simulink library "inp"  (Simulink 8.2)
settings: Solver=ode45  SolverMode=SingleTasking  StartTime=0.0  StopTime=10.0  RelTol=1e-3
undefined symbols (not defined in the file or in its folder): C_TICK_TIME_S, ENUM_FAULTSTATE_FAIL, ENUM_FAULTSTATE_INDETERMINATE, ENUM_FAULTSTATE_PASS, ENUM_KEYSWITCH_ON, FALSE, KINP_ABS1CANCommuOk_flg_ovrdflg, KINP_ABS1CANCommuOk_flg_ovrdval, KINP_ABS1CANPeriodTime_s, KINP_ABS1CANRolCntOk_flg_ovrdflg, KINP_ABS1CANRolCntOk_flg_ovrdval, KINP_ABS2CANChkSumOk_flg_ovrdflg, KINP_ABS2CANChkSumOk_flg_ovrdval, KINP_ABS2CANCommuOk_flg_ovrdflg, KINP_ABS2CANCommuOk_flg_ovrdval, KINP_ABS2CANPeriodTime_s, KINP_ABS2CANRolCntOk_flg_ovrdflg, KINP_ABS2CANRolCntOk_flg_ovrdval, KINP_ABS3CANCommuOk_flg_ovrdflg, KINP_ABS3CANCommuOk_flg_ovrdval, KINP_ABS4CANCommuOk_flg_ovrdflg, KINP_ABS4CANCommuOk_flg_ovrdval, KINP_ABSActive_flg_defval, KINP_ABSActive_flg_invalid, KINP_ABSActive_flg_ovrdflg, KINP_ABSActive_flg_ovrdval, KINP_ABSActive_flg_setvalid, KINP_ABSActive_flg_srvcflg, KINP_ABSActive_flg_srvcval, KINP_ABSCANAlive_flg_ovrdflg, KINP_ABSCANAlive_flg_ovrdval, KINP_ABSCANCommuOk_flg_ovrdflg, KINP_ABSCANCommuOk_flg_ovrdval, KINP_ABSCANValidOk_flg_ovrdflg, KINP_ABSCANValidOk_flg_ovrdval, KINP_ABSFault_flg_defval, KINP_ABSFault_flg_invalid, KINP_ABSFault_flg_ovrdflg, KINP_ABSFault_flg_ovrdval, KINP_ABSFault_flg_setvalid, KINP_ABSFault_flg_srvcflg, KINP_ABSFault_flg_srvcval, KINP_AC1CANCommuOk_flg_ovrdflg, KINP_AC1CANCommuOk_flg_ovrdval, KINP_ACCANAlive_flg_ovrdflg, KINP_ACCANAlive_flg_ovrdval, KINP_ACCANCommuOk_flg_ovrdflg, KINP_ACCANCommuOk_flg_ovrdval, KINP_ACCANValidOk_flg_ovrdflg, KINP_ACCANValidOk_flg_ovrdval, KINP_ACCCancelH_flg_defval, KINP_ACCCancelH_flg_invalid, KINP_ACCCancelH_flg_ovrdflg, KINP_ACCCancelH_flg_ovrdval, KINP_ACCCancelH_flg_setvalid, KINP_ACCCancelH_flg_srvcflg, KINP_ACCCancelH_flg_srvcval, KINP_AccelPedalPosn_pct_defval, KINP_AccelPedalPosn_pct_gain, KINP_AccelPedalPosn_pct_invalid ... (+1866)
libraries: inp x192, hcu_lib x34, simulink x33
(part 1 of 21)
settings Model: Creator=WX  LastModifiedBy=13192

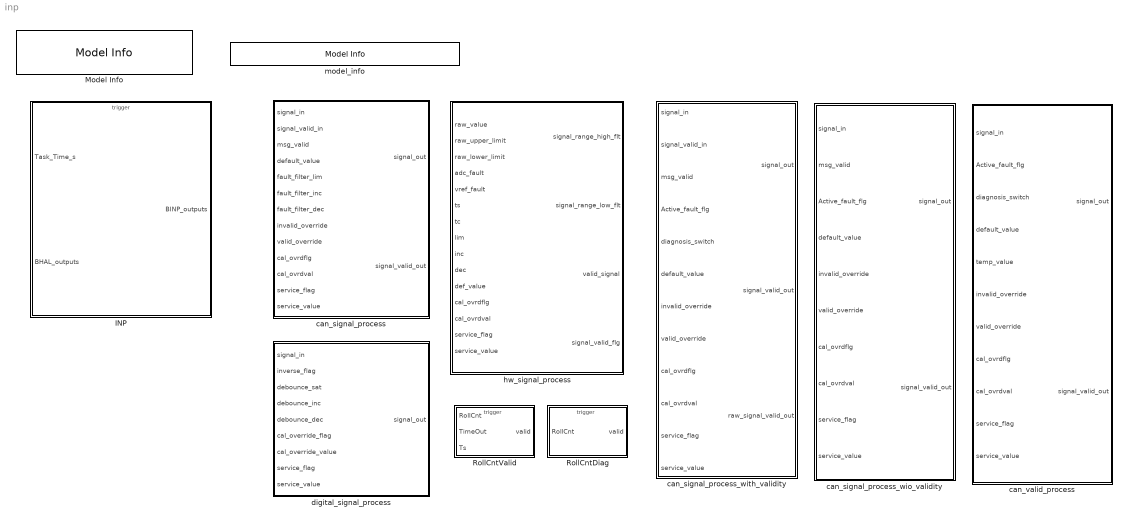
== system inp ==
  INP : Subsystem
      settings: RTWSystemCode=Nonreusable function  RTWFcnNameOpts=Use subsystem name  RTWFileNameOpts=Use subsystem name
  Model Info : simulink/Model-Wide Utilities/Model Info  InitialBlockCM=None  BlockCM=None  Frame=on  DisplayStringWithTags=|  HorizontalTextAlignment=Left  LeftAlignmentValue=0.02  SourceBlockDiagram=inp  TagMaxNumber=20
      | Model Version        : %<ModelVersion>
      | Creator                   : %<Creator>
      | Last Modified by    : %<LastModifiedBy>
  RollCntDiag : Subsystem
  RollCntValid : Subsystem
  can_signal_process : Subsystem
  can_signal_process_wio_validity : Subsystem
  can_signal_process_with_validity : Subsystem
  can_valid_process : Subsystem
  digital_signal_process : Subsystem
  hw_signal_process : Subsystem
  model_info : simulink/Model-Wide Utilities/Model Info  InitialBlockCM=None  BlockCM=None  Frame=on  DisplayStringWithTags=$Id: hcu_lib.mdl 289 2016-04-11 06:01:08Z chimotsai $  HorizontalTextAlignment=Center  LeftAlignmentValue=0.5  SourceBlockDiagram=inp  TagMaxNumber=20

== system inp/INP ==
  Task_Time_s : Inport
  BHAL_outputs : Inport  Port=2  OutDataTypeStr=Bus: BHAL_outputs
  trig_inp : TriggerPort  TriggerType=function-call
  ABS_signals : Subsystem
  AC_signals : Subsystem
  BCM_signals : Subsystem
  CAN_Message_Valid_Proc : Subsystem
  CAN_Signals_Protected : Subsystem
  CSW_signals : Subsystem
  ECM_signals : Subsystem
  EPB_signals : Subsystem
  EPM_signals : Subsystem
  EPS_signals : Subsystem
  HMI_signals : Subsystem
  HWIO_signals : Subsystem
  InputSignals : Subsystem
  LLSW_signals : Subsystem
  METER_signals : Subsystem
  TCM_signals : Subsystem
  VCU_signals : Subsystem
  BINP_outputs : Outport  OutDataTypeStr=Bus: BINP_outputs
  wires:
  [CANvalid_bus] -> ECM_signals/2(CANvalid_bus)
  CAN_Signals_Protected/1(ProtCC_bus) -> [ProtCC_bus]
  [BHAL_outputs] -> CAN_Signals_Protected/1(BHAL_outputs)
  [Task_Time_s] -> CAN_Message_Valid_Proc/2(Task_Time_s)
  CAN_Message_Valid_Proc/1(CANvalid_bus) -> [CANvalid_bus]
  [BHAL_outputs] -> LLSW_signals/1(BHAL_outputs)
  [Task_Time_s] -> InputSignals/15(Task_Time_s)
  LLSW_signals/1(LLSW_signal_bus) -> InputSignals/14(LLSW_signal_bus)
  [Task_Time_s] -> HWIO_signals/2(Task_Time_s)
  [BHAL_outputs] -> HWIO_signals/1(BHAL_outputs)
  [BHAL_outputs] -> EPM_signals/1(BHAL_outputs)
  [BHAL_outputs] -> EPS_signals/1(BHAL_outputs)
  [BHAL_outputs] -> ABS_signals/1(BHAL_outputs)
  [BHAL_outputs] -> BCM_signals/1(BHAL_outputs)
  [BHAL_outputs] -> TCM_signals/1(BHAL_outputs)
  Task_Time_s/1 -> [Task_Time_s]
  [BHAL_outputs] -> ECM_signals/1(BHAL_outputs)
  HWIO_signals/1(HWIO_signal_bus) -> InputSignals/13(HWIO_signal_bus)
  EPM_signals/1(EPM_signal_bus) -> InputSignals/12(EPM_signal_bus)
  EPS_signals/1(EPS_signal_bus) -> InputSignals/5(EPS_signal_bus)
  ABS_signals/1(ABS_signal_bus) -> InputSignals/4(ABS_signal_bus)
  BCM_signals/1(BCM_signal_bus) -> InputSignals/3(BCM_signal_bus)
  TCM_signals/1(TCM_signal_bus) -> InputSignals/2(TCM_signal_bus)
  ECM_signals/1(ECM_signal_bus) -> InputSignals/1(ECM_signal_bus)
  InputSignals/1(BINP_outputs) -> BINP_outputs/1
  BHAL_outputs/1 -> [BHAL_outputs], CAN_Message_Valid_Proc/1(BHAL_outputs)
  [CANvalid_bus] -> TCM_signals/2(CANvalid_bus)
  [CANvalid_bus] -> BCM_signals/2(CANvalid_bus)
  [CANvalid_bus] -> ABS_signals/2(CANvalid_bus)
  [CANvalid_bus] -> CAN_Signals_Protected/2(CANvalid_bus)
  [CANvalid_bus] -> EPS_signals/2(CANvalid_bus)
  [CANvalid_bus] -> EPM_signals/2(CANvalid_bus)
  [CANvalid_bus] -> InputSignals/17(CANvalid_bus)
  [ProtCC_bus] -> InputSignals/16(ProtCC_bus)
  [BHAL_outputs] -> METER_signals/1(BHAL_outputs)
  [CANvalid_bus] -> METER_signals/2(CANvalid_bus)
  [BHAL_outputs] -> AC_signals/1(BHAL_outputs)
  [CANvalid_bus] -> AC_signals/2(CANvalid_bus)
  [BHAL_outputs] -> EPB_signals/1(BHAL_outputs)
  [CANvalid_bus] -> EPB_signals/2(CANvalid_bus)
  METER_signals/1(METER_signal_bus) -> InputSignals/6(METER_signal_bus)
  AC_signals/1(AC_signal_bus) -> InputSignals/7(AC_signal_bus)
  EPB_signals/1(EPB_signal_bus) -> InputSignals/8(EPB_signal_bus)
  [BHAL_outputs] -> VCU_signals/1(BHAL_outputs)
  [CANvalid_bus] -> VCU_signals/2(CANvalid_bus)
  VCU_signals/1(VCU_signal_bus) -> InputSignals/10(VCU_signal_bus)
  [BHAL_outputs] -> HMI_signals/1(BHAL_outputs)
  [CANvalid_bus] -> HMI_signals/2(CANvalid_bus)
  HMI_signals/1(HMI_signal_bus) -> InputSignals/11(HMI_signal_bus)
  [BHAL_outputs] -> CSW_signals/1(BHAL_outputs)
  [CANvalid_bus] -> CSW_signals/2(CANvalid_bus)
  CSW_signals/1(CSW_signal_bus) -> InputSignals/9(CSW_signal_bus)

== system inp/RollCntDiag ==
  RollCnt : Inport
  Sampler : TriggerPort  TriggerType=function-call
  Constant : Constant  Value=FALSE
  Constant1 : Constant  Value=TRUE
  Logical Operator : Logic  Operator=NOT  AllPortsSameDT=off  OutDataTypeStr=boolean
  Relational Operator1 : RelationalOperator  Operator===  OutDataTypeStr=boolean
  Switch : Switch  Criteria=u2 ~= 0
  Unit Delay1 : UnitDelay  SampleTime=-1
  Unit Delay2 : UnitDelay  InitialCondition=TRUE  SampleTime=-1
  valid : Outport
  wires:
  Relational Operator1/1 -> Logical Operator/1
  Unit Delay1/1 -> Relational Operator1/1
  RollCnt/1 -> Relational Operator1/2, Unit Delay1/1
  Logical Operator/1 -> Switch/3
  Switch/1 -> valid/1
  Constant/1 -> Unit Delay2/1
  Unit Delay2/1 -> Switch/2
  Constant1/1 -> Switch/1

== system inp/RollCntValid ==
  RollCnt : Inport
  TimeOut : Inport  Port=2
  Ts : Inport  Port=3
  Sampler : TriggerPort  TriggerType=function-call
  Add : Sum
  RESET : Constant  Value=0  OutDataTypeStr=single
  Relational Operator1 : RelationalOperator  Operator===  OutDataTypeStr=boolean
  Relational Operator2 : RelationalOperator  Operator=<  OutDataTypeStr=boolean
  Switch : Switch  Criteria=u2 ~= 0
  Unit Delay : UnitDelay  SampleTime=-1
  Unit Delay1 : UnitDelay  SampleTime=-1
  valid : Outport
  wires:
  Ts/1 -> Add/2
  Add/1 -> Switch/1
  Unit Delay/1 -> Add/1
  RollCnt/1 -> Unit Delay1/1, Relational Operator1/2
  Unit Delay1/1 -> Relational Operator1/1
  Switch/1 -> Unit Delay/1, Relational Operator2/1
  Relational Operator1/1 -> Switch/2
  RESET/1 -> Switch/3
  TimeOut/1 -> Relational Operator2/2
  Relational Operator2/1 -> valid/1

== system inp/can_signal_process ==
  signal_in : Inport
  signal_valid_in : Inport  Port=2
  msg_valid : Inport  Port=3
  default_value : Inport  Port=4
  fault_filter_lim : Inport  Port=5
  fault_filter_inc : Inport  Port=6
  fault_filter_dec : Inport  Port=7
  invalid_override : Inport  Port=8
  valid_override : Inport  Port=9
  cal_ovrdflg : Inport  Port=10
  cal_ovrdval : Inport  Port=11
  service_flag : Inport  Port=12
  service_value : Inport  Port=13
  Logical Operator1 : Logic  Operator=NOT  AllPortsSameDT=off  OutDataTypeStr=boolean
  Logical Operator2 : Logic  AllPortsSameDT=off  OutDataTypeStr=boolean
  SRS_HCU_INP_002 : simulink/Model-Wide Utilities/DocBlock  DocumentType=Text
  override signal : hcu_lib/override signal
  signal validity checking : hcu_lib/sig_valid_check
  signal_out : Outport
  signal_valid_out : Outport  Port=2
  wires:
  override signal/2 -> signal_valid_out/1
  override signal/1 -> signal_out/1
  msg_valid/1 -> Logical Operator2/2
  signal_in/1 -> signal validity checking/1
  signal_valid_in/1 -> Logical Operator2/1
  Logical Operator2/1 -> signal validity checking/2
  invalid_override/1 -> signal validity checking/3
  Logical Operator1/1 -> override signal/1  "signal_valid_flg_raw"
  service_flag/1 -> override signal/3
  service_value/1 -> override signal/4
  cal_ovrdflg/1 -> override signal/5
  cal_ovrdval/1 -> override signal/6
  valid_override/1 -> signal validity checking/4
  default_value/1 -> signal validity checking/5
  fault_filter_lim/1 -> signal validity checking/6
  fault_filter_inc/1 -> signal validity checking/7
  fault_filter_dec/1 -> signal validity checking/8
  signal validity checking/1 -> Logical Operator1/1  "fault_flg"
  signal validity checking/2 -> override signal/2  "valid_flg"

== system inp/can_signal_process_wio_validity ==
  signal_in : Inport
  msg_valid : Inport  Port=2
  Active_fault_flg : Inport  Port=3
  default_value : Inport  Port=4
  invalid_override : Inport  Port=5
  valid_override : Inport  Port=6
  cal_ovrdflg : Inport  Port=7
  cal_ovrdval : Inport  Port=8
  service_flag : Inport  Port=9
  service_value : Inport  Port=10
  CAN signal validity checking no diag : hcu_lib/CAN signal validity checking no diag
  Logical Operator1 : Logic  Operator=NOT  AllPortsSameDT=off  OutDataTypeStr=boolean
  override signal : hcu_lib/override signal
  signal_out : Outport
  signal_valid_out : Outport  Port=2
  wires:
  override signal/2 -> signal_valid_out/1
  override signal/1 -> signal_out/1
  msg_valid/1 -> CAN signal validity checking no diag/2
  signal_in/1 -> CAN signal validity checking no diag/1
  invalid_override/1 -> CAN signal validity checking no diag/3
  Logical Operator1/1 -> override signal/1  "signal_valid_flg_raw"
  service_flag/1 -> override signal/3
  service_value/1 -> override signal/4
  cal_ovrdflg/1 -> override signal/5
  cal_ovrdval/1 -> override signal/6
  valid_override/1 -> CAN signal validity checking no diag/4
  default_value/1 -> CAN signal validity checking no diag/5
  Active_fault_flg/1 -> CAN signal validity checking no diag/6
  CAN signal validity checking no diag/2 -> override signal/2  "signal_proc"
  CAN signal validity checking no diag/1 -> Logical Operator1/1  "fault_flag"

== system inp/can_signal_process_with_validity ==
  signal_in : Inport
  signal_valid_in : Inport  Port=2
  msg_valid : Inport  Port=3
  Active_fault_flg : Inport  Port=4
  diagnosis_switch : Inport  Port=5
  default_value : Inport  Port=6
  invalid_override : Inport  Port=7
  valid_override : Inport  Port=8
  cal_ovrdflg : Inport  Port=9
  cal_ovrdval : Inport  Port=10
  service_flag : Inport  Port=11
  service_value : Inport  Port=12
  CAN signal validity checking : hcu_lib/CAN signal validity checking
  Logical Operator1 : Logic  Operator=NOT  AllPortsSameDT=off  OutDataTypeStr=boolean
  Logical Operator2 : Logic  Operator=NOT  AllPortsSameDT=off  OutDataTypeStr=boolean
  override signal : hcu_lib/override signal
  signal_out : Outport
  signal_valid_out : Outport  Port=2
  raw_signal_valid_out : Outport  Port=3
  wires:
  CAN signal validity checking/1 -> Logical Operator2/1
  CAN signal validity checking/2 -> Logical Operator1/1  "fault_flag"
  CAN signal validity checking/3 -> override signal/2  "signal_proc"
  Active_fault_flg/1 -> CAN signal validity checking/7
  default_value/1 -> CAN signal validity checking/6
  valid_override/1 -> CAN signal validity checking/5
  cal_ovrdval/1 -> override signal/6
  cal_ovrdflg/1 -> override signal/5
  service_value/1 -> override signal/4
  service_flag/1 -> override signal/3
  Logical Operator1/1 -> override signal/1  "signal_valid_flg_raw"
  invalid_override/1 -> CAN signal validity checking/4
  signal_valid_in/1 -> CAN signal validity checking/2
  signal_in/1 -> CAN signal validity checking/1
  msg_valid/1 -> CAN signal validity checking/3
  override signal/1 -> signal_out/1
  override signal/2 -> signal_valid_out/1
  diagnosis_switch/1 -> CAN signal validity checking/8
  Logical Operator2/1 -> raw_signal_valid_out/1

== system inp/can_valid_process ==
  signal_in : Inport
  Active_fault_flg : Inport  Port=2
  diagnosis_switch : Inport  Port=3
  default_value : Inport  Port=4
  temp_value : Inport  Port=5
  invalid_override : Inport  Port=6
  valid_override : Inport  Port=7
  cal_ovrdflg : Inport  Port=8
  cal_ovrdval : Inport  Port=9
  service_flag : Inport  Port=10
  service_value : Inport  Port=11
  Logical Operator1 : Logic  Operator=NOT  AllPortsSameDT=off  OutDataTypeStr=boolean
  override signal : hcu_lib/override signal
  signal validity checking with temp value : hcu_lib/signal validity checking with temp value
  signal_out : Outport
  signal_valid_out : Outport  Port=2
  wires:
  signal validity checking with temp value/2 -> override signal/2  "signal_proc"
  signal validity checking with temp value/1 -> Logical Operator1/1  "fault_flg"
  Active_fault_flg/1 -> signal validity checking with temp value/7
  default_value/1 -> signal validity checking with temp value/6
  valid_override/1 -> signal validity checking with temp value/4
  cal_ovrdval/1 -> override signal/6
  cal_ovrdflg/1 -> override signal/5
  service_value/1 -> override signal/4
  service_flag/1 -> override signal/3
  Logical Operator1/1 -> override signal/1  "signal_valid_flg_raw"
  invalid_override/1 -> signal validity checking with temp value/3
  signal_in/1 -> signal validity checking with temp value/2, signal validity checking with temp value/1
  override signal/1 -> signal_out/1
  override signal/2 -> signal_valid_out/1
  temp_value/1 -> signal validity checking with temp value/5
  diagnosis_switch/1 -> signal validity checking with temp value/8

== system inp/digital_signal_process ==
  signal_in : Inport
  inverse_flag : Inport  Port=2
  debounce_sat : Inport  Port=3
  debounce_inc : Inport  Port=4
  debounce_dec : Inport  Port=5
  cal_override_flag : Inport  Port=6
  cal_override_value : Inport  Port=7
  service_flag : Inport  Port=8
  service_value : Inport  Port=9
  Ground : Ground
  Logical Operator : Logic  Operator=NOT  Inputs=1  AllPortsSameDT=off  OutDataTypeStr=boolean
  Switch1 : Switch  Criteria=u2 ~= 0  Threshold=0.5
  Terminator : Terminator
  Terminator1 : Terminator
  override_signal : hcu_lib/override signal
  resetting fault filter : hcu_lib/fault_filter_reset
  signal_out : Outport  OutDataTypeStr=boolean
  wires:
  debounce_dec/1 -> resetting fault filter/4
  debounce_inc/1 -> resetting fault filter/3
  debounce_sat/1 -> resetting fault filter/2
  override_signal/1 -> signal_out/1
  resetting fault filter/2 -> Terminator/1
  override_signal/2 -> Terminator1/1
  resetting fault filter/1 -> override_signal/2
  Ground/1 -> override_signal/1
  service_flag/1 -> override_signal/3
  service_value/1 -> override_signal/4
  cal_override_flag/1 -> override_signal/5
  cal_override_value/1 -> override_signal/6
  inverse_flag/1 -> Switch1/2
  signal_in/1 -> Switch1/3, Logical Operator/1
  Switch1/1 -> resetting fault filter/1
  Logical Operator/1 -> Switch1/1

== system inp/hw_signal_process ==
  raw_value : Inport
  raw_upper_limit : Inport  Port=2
  raw_lower_limit : Inport  Port=3
  adc_fault : Inport  Port=4
  vref_fault : Inport  Port=5
  ts : Inport  Port=6
  tc : Inport  Port=7
  lim : Inport  Port=8
  inc : Inport  Port=9
  dec : Inport  Port=10
  def_value : Inport  Port=11
  cal_ovrdflg : Inport  Port=12
  cal_ovrdval : Inport  Port=13
  service_flag : Inport  Port=14
  service_value : Inport  Port=15
  Logical Operator2 : Logic  Operator=NOT  AllPortsSameDT=off  OutDataTypeStr=boolean
  Relational Operator : RelationalOperator  Operator=<  OutDataTypeStr=boolean
  Relational Operator1 : RelationalOperator  Operator=<  OutDataTypeStr=boolean
  SRS_HCU_INP_004 : simulink/Model-Wide Utilities/DocBlock  DocumentType=Text
  override signal : hcu_lib/override signal
  sig_valid_check : Subsystem  RTWFcnName=sig_valid_check
      settings: RTWFcnNameOpts=User specified
  signal_filter : Subsystem
  signal_range_high_flt : Outport
  signal_range_low_flt : Outport  Port=2
  valid_signal : Outport  Port=3
  signal_valid_flg : Outport  Port=4
  wires:
  Logical Operator2/1 -> override signal/1
  ts/1 -> signal_filter/3(Ts)
  tc/1 -> signal_filter/2(Tc)
  raw_value/1 -> [raw_value], signal_filter/1(raw_input)
  Relational Operator/1 -> sig_valid_check/4(range_high_ifault_flg)
  [raw_value] -> Relational Operator1/1, Relational Operator/2
  def_value/1 -> sig_valid_check/9(def_value)
  dec/1 -> sig_valid_check/8(dec)
  inc/1 -> sig_valid_check/7(inc)
  lim/1 -> sig_valid_check/6(lim)
  raw_lower_limit/1 -> Relational Operator1/2
  raw_upper_limit/1 -> Relational Operator/1
  sig_valid_check/3(fault_flg) -> Logical Operator2/1
  signal_filter/1(raw_input_filt) -> sig_valid_check/1(input_signal_filt)
  Relational Operator1/1 -> sig_valid_check/5(range_low_ifault_flg)
  sig_valid_check/1(range_high_flt) -> signal_range_high_flt/1
  sig_valid_check/2(range_low_flt) -> signal_range_low_flt/1
  service_flag/1 -> override signal/3
  service_value/1 -> override signal/4
  cal_ovrdflg/1 -> override signal/5
  cal_ovrdval/1 -> override signal/6
  sig_valid_check/4(valid_signal) -> override signal/2
  override signal/1 -> valid_signal/1
  override signal/2 -> signal_valid_flg/1
  adc_fault/1 -> sig_valid_check/2(adc_fault)
  vref_fault/1 -> sig_valid_check/3(vref_fault)

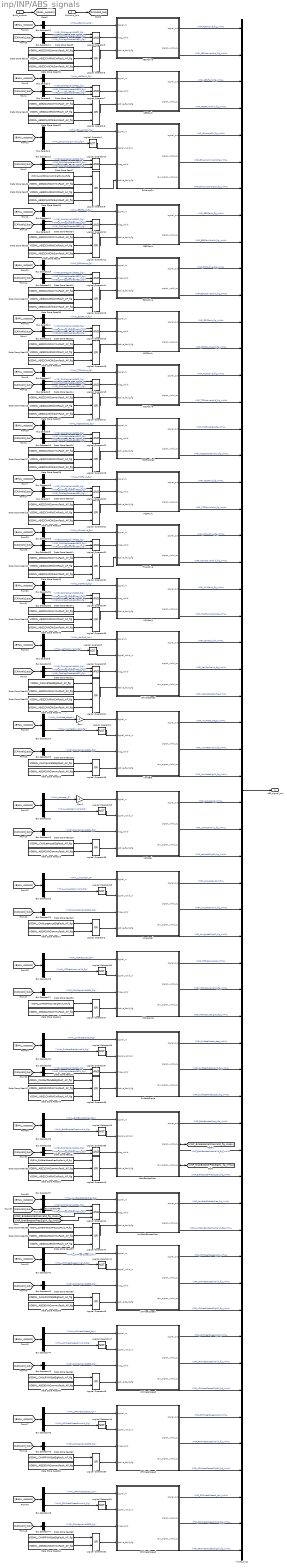
== system inp/INP/ABS_signals ==
  BHAL_outputs : Inport  OutDataTypeStr=Bus: BHAL_outputs
  CANvalid_bus : Inport  Port=2
  ABSActive : Subsystem
      settings: RTWSystemCode=Inline
  ABSFault : Subsystem
      settings: RTWSystemCode=Inline
  BrakBoosterPres : Subsystem
      settings: RTWSystemCode=Inline
  BrkLampOn : Subsystem
      settings: RTWSystemCode=Inline
  Bus Selector1 : BusSelector  OutputSignals=VHAL_ABSFault_flg
  Bus Selector10 : BusSelector  OutputSignals=VHAL_ABSActive_flg
  Bus Selector{3,5,7,9,11..12,16,18,20..21,30,32} (12 alike) : BusSelector  OutputSignals=VINP_CANMsgValidABS2_flg,VINP_CANMsgUpdatedABS2_flg,VINP_CANMsgCheckedABS2_flg
  Bus Selector{13,23,25,27} (4 alike) : BusSelector  OutputSignals=VINP_CANMsgValidABS4_flg
  Bus Selector14 : BusSelector  OutputSignals=VHAL_EBDFault_flg
  Bus Selector15 : BusSelector  OutputSignals=VHAL_ESCActive_flg
  Bus Selector17 : BusSelector  OutputSignals=VHAL_TCSActive_flg
  Bus Selector19 : BusSelector  OutputSignals=VHAL_TCSFault_flg
  Bus Selector2 : BusSelector  OutputSignals=VHAL_BrkLampOn_flg,VHAL_BrkLampSigInvalid_flg
  Bus Selector22 : BusSelector  OutputSignals=VHAL_LatAccel_G,VHAL_LatAccelInvalid_flg
  Bus Selector24 : BusSelector  OutputSignals=VHAL_YawRate_degps,VHAL_YawRateInvalid_flg
  Bus Selector26 : BusSelector  OutputSignals=VHAL_LongAccel_G,VHAL_LongAccelInvalid_flg
  Bus Selector28 : BusSelector  OutputSignals=VHAL_MCPressure_bar,VHAL_MCPressureInvalid_flg
  Bus Selector29 : BusSelector  OutputSignals=VHAL_HSAActive_flg
  Bus Selector31 : BusSelector  OutputSignals=VHAL_HSAFault_flg
  Bus Selector33 : BusSelector  OutputSignals=VINP_CANMsgValidABS1_flg,VINP_CANMsgUpdatedABS1_flg
  Bus Selector34 : BusSelector  OutputSignals=VHAL_SurfaceGrade_deg,VHAL_SurfaceGradeInvalid_flg
  Bus Selector35 : BusSelector  OutputSignals=VINP_CANMsgValidABS1_flg,VINP_CANMsgUpdatedABS1_flg
  Bus Selector36 : BusSelector  OutputSignals=VHAL_BrakBoosterPres_kPa,VHAL_BrakBoosterPresInvalid_flg
  Bus Selector37 : BusSelector  OutputSignals=VHAL_LowBrakBoosterPres_flg
  Bus Selector38 : BusSelector  OutputSignals=VINP_CANMsgValidABS1_flg,VINP_CANMsgUpdatedABS1_flg
  Bus Selector4 : BusSelector  OutputSignals=VHAL_ESCFault_flg
  Bus Selector{40,43,45,47} (4 alike) : BusSelector  OutputSignals=VINP_CANMsgValidABS3_flg
  Bus Selector42 : BusSelector  OutputSignals=VHAL_LFWheelSpeed_kph,VHAL_LFWheelSpeedInvalid_flg
  Bus Selector44 : BusSelector  OutputSignals=VHAL_LRWheelSpeed_kph,VHAL_LRWheelSpeedInvalid_flg
  Bus Selector46 : BusSelector  OutputSignals=VHAL_RFWheelSpeed_kph,VHAL_RFWheelSpeedInvalid_flg
  Bus Selector48 : BusSelector  OutputSignals=VHAL_RRWheelSpeed_kph,VHAL_RRWheelSpeedInvalid_flg
  Bus Selector6 : BusSelector  OutputSignals=VHAL_TCSEnabled_flg
  Bus Selector8 : BusSelector  OutputSignals=VHAL_VehSpd_kph,VHAL_VehSpdInvalid_flg
  Data Store Read{1..2,5,8,11,13,15,17,19..20,42,45} (12 alike) : DataStoreRead  DataStoreName=VDDML_ABS2CANRolCntFault_AF_flg
  Data Store Read{3..4,6,10,12,14,16,18,21,34,41,44} (12 alike) : DataStoreRead  DataStoreName=VDDML_ABS2CANCommuFault_AF_flg
  Data Store Read22 : DataStoreRead  DataStoreName=VDDML_CANVehSpdSigFault_AF_flg
  Data Store Read{23..25,27} (4 alike) : DataStoreRead  DataStoreName=VDDML_ABS4CANCommuFault_AF_flg
  Data Store Read26 : DataStoreRead  DataStoreName=VDDML_CANYawRateSigFault_AF_flg
  Data Store Read28 : DataStoreRead  DataStoreName=VDDML_CANLatAccelSigFault_AF_flg
  Data Store Read29 : DataStoreRead  DataStoreName=VDDML_CANLongAccelSigFault_AF_flg
  Data Store Read30 : DataStoreRead  DataStoreName=VDDML_CANMCPressureSigFault_AF_flg
  Data Store Read{9,31..33,35..40,43,46} (12 alike) : DataStoreRead  DataStoreName=VDDML_ABS2CANChkSumFault_AF_flg
  Data Store Read47 : DataStoreRead  DataStoreName=VDDML_ABS1CANCommuFault_AF_flg
  Data Store Read48 : DataStoreRead  DataStoreName=VDDML_CANSurfGradeSigFault_AF_flg
  Data Store Read49 : DataStoreRead  DataStoreName=VDDML_ABS1CANRolCntFault_AF_flg
  Data Store Read50 : DataStoreRead  DataStoreName=VDDML_ABS1CANCommuFault_AF_flg
  Data Store Read51 : DataStoreRead  DataStoreName=VDDML_CANBrakBoostPresSigFault_AF_flg
  Data Store Read52 : DataStoreRead  DataStoreName=VDDML_ABS1CANRolCntFault_AF_flg
  Data Store Read53 : DataStoreRead  DataStoreName=VDDML_CANBrakBoostPresSigFault_AF_flg
  Data Store Read54 : DataStoreRead  DataStoreName=VDDML_ABS1CANCommuFault_AF_flg
  Data Store Read55 : DataStoreRead  DataStoreName=VDDML_ABS1CANRolCntFault_AF_flg
  Data Store Read{58,60,62,64} (4 alike) : DataStoreRead  DataStoreName=VDDML_ABS3CANCommuFault_AF_flg
  Data Store Read59 : DataStoreRead  DataStoreName=VDDML_CANLFWhlSpdSigFault_AF_flg
  Data Store Read61 : DataStoreRead  DataStoreName=VDDML_CANLRWhlSpdSigFault_AF_flg
  Data Store Read63 : DataStoreRead  DataStoreName=VDDML_CANRFWhlSpdSigFault_AF_flg
  Data Store Read65 : DataStoreRead  DataStoreName=VDDML_CANRRWhlSpdSigFault_AF_flg
  Data Store Read7 : DataStoreRead  DataStoreName=VDDML_CANBrkLampOnSigFault_AF_flg
  EBDFault : Subsystem
      settings: RTWSystemCode=Inline
  ESCActive : Subsystem
      settings: RTWSystemCode=Inline
  ESCFault : Subsystem
      settings: RTWSystemCode=Inline
  Gain : Gain  Gain=-1
  Gain1 : Gain  Gain=-1
  HSAActive : Subsystem
      settings: RTWSystemCode=Inline
  HSAFault : Subsystem
      settings: RTWSystemCode=Inline
  LFWheelSpeed : Subsystem
      settings: RTWSystemCode=Inline
  LRWheelSpeed : Subsystem
      settings: RTWSystemCode=Inline
  LatAccel : Subsystem
      settings: RTWSystemCode=Inline
  Logical Operator{1,3..4,25,28..30,36,44,47,49,51} (12 alike) : Logic  Tag=struct('inputs','1','comment',struct('text','','placement',1),'logdata',struct('maxnumvals',inf,'plotchannels',[],'stepsize',0,'downsampling',1,'loggingmode',3,'channels',1),'operator','NOT','output',struct('class','default','type','Bool8','description','','address','','unit','','name','$S_$B'),'date',731430.46749816)  Operator=NOT  Inputs=1
  Logical Operator{7,9..10,12,14,16,18,20,22..23,32,34} (12 alike) : Logic  Tag=struct('inputs','1','comment',struct('text','','placement',1),'logdata',struct('maxnumvals',inf,'plotchannels',[],'stepsize',0,'downsampling',1,'loggingmode',3,'channels',1),'operator','NOT','output',struct('class','default','type','Bool8','description','','address','','unit','','name','$S_$B'),'date',731430.46749816)  Inputs=3
  Logical Operator{5..6,11,13,15,17,19,21,31,33,38,40..41} (13 alike) : Logic  Tag=struct('inputs','1','comment',struct('text','','placement',1),'logdata',struct('maxnumvals',inf,'plotchannels',[],'stepsize',0,'downsampling',1,'loggingmode',3,'channels',1),'operator','NOT','output',struct('class','default','type','Bool8','description','','address','','unit','','name','$S_$B'),'date',731430.46749816)  Operator=OR  Inputs=3
  Logical Operator{2,26..27,35,46,48,50,52} (8 alike) : Logic  Tag=struct('inputs','1','comment',struct('text','','placement',1),'logdata',struct('maxnumvals',inf,'plotchannels',[],'stepsize',0,'downsampling',1,'loggingmode',3,'channels',1),'operator','NOT','output',struct('class','default','type','Bool8','description','','address','','unit','','name','$S_$B'),'date',731430.46749816)  Operator=OR
  Logical Operator24 : Logic  Tag=struct('inputs','1','comment',struct('text','','placement',1),'logdata',struct('maxnumvals',inf,'plotchannels',[],'stepsize',0,'downsampling',1,'loggingmode',3,'channels',1),'operator','NOT','output',struct('class','default','type','Bool8','description','','address','','unit','','name','$S_$B'),'date',731430.46749816)  Operator=OR  Inputs=4
  Logical Operator37 : Logic  Tag=struct('inputs','1','comment',struct('text','','placement',1),'logdata',struct('maxnumvals',inf,'plotchannels',[],'stepsize',0,'downsampling',1,'loggingmode',3,'channels',1),'operator','NOT','output',struct('class','default','type','Bool8','description','','address','','unit','','name','$S_$B'),'date',731430.46749816)
  Logical Operator39 : Logic  Tag=struct('inputs','1','comment',struct('text','','placement',1),'logdata',struct('maxnumvals',inf,'plotchannels',[],'stepsize',0,'downsampling',1,'loggingmode',3,'channels',1),'operator','NOT','output',struct('class','default','type','Bool8','description','','address','','unit','','name','$S_$B'),'date',731430.46749816)
  Logical Operator42 : Logic  Tag=struct('inputs','1','comment',struct('text','','placement',1),'logdata',struct('maxnumvals',inf,'plotchannels',[],'stepsize',0,'downsampling',1,'loggingmode',3,'channels',1),'operator','NOT','output',struct('class','default','type','Bool8','description','','address','','unit','','name','$S_$B'),'date',731430.46749816)  Inputs=4
  Logical Operator8 : Logic  Tag=struct('inputs','1','comment',struct('text','','placement',1),'logdata',struct('maxnumvals',inf,'plotchannels',[],'stepsize',0,'downsampling',1,'loggingmode',3,'channels',1),'operator','NOT','output',struct('class','default','type','Bool8','description','','address','','unit','','name','$S_$B'),'date',731430.46749816)  Operator=OR  Inputs=4
  LongAccel : Subsystem
      settings: RTWSystemCode=Inline
  LowBrakBoosterPres : Subsystem
      settings: RTWSystemCode=Inline
  MCPressure : Subsystem
      settings: RTWSystemCode=Inline
  RFWheelSpeed : Subsystem
      settings: RTWSystemCode=Inline
  RRWheelSpeed : Subsystem
      settings: RTWSystemCode=Inline
  SurfaceGrade : Subsystem
      settings: RTWSystemCode=Inline
  TCSActive : Subsystem
      settings: RTWSystemCode=Inline
  TCSEnabled : Subsystem
      settings: RTWSystemCode=Inline
  TCSFault : Subsystem
      settings: RTWSystemCode=Inline
  VehicleSpeed : Subsystem
      settings: RTWSystemCode=Inline
  YawRate : Subsystem
      settings: RTWSystemCode=Inline
  inputs_bus2 : BusCreator  Inputs=58
  ABS_signal_bus : Outport
  wires:
  inputs_bus2/1 -> ABS_signal_bus/1
  BHAL_outputs/1 -> [BHAL_outputs]
  Bus Selector11/1 -> Logical Operator9/1  "<VINP_CANMsgValidABS2_flg>"
  [CANvalid_bus] -> Bus Selector11/1
  Bus Selector10/1 -> ABSActive/1(signal_in)  "<VHAL_ABSActive_flg>"
  [BHAL_outputs] -> Bus Selector10/1
  Logical Operator5/1 -> ABSActive/3(Active_fault_flg)
  ABSActive/1(signal_out) -> inputs_bus2/1  "VINP_ABSActive_flg_InHAL"
  ABSActive/2(signal_valid_out) -> inputs_bus2/2  "VINP_ABSActiveValid_flg_InHAL"
  Bus Selector1/1 -> ABSFault/1(signal_in)  "<VHAL_ABSFault_flg>"
  [BHAL_outputs] -> Bus Selector1/1
  ABSFault/1(signal_out) -> inputs_bus2/3  "VINP_ABSFault_flg_InHAL"
  ABSFault/2(signal_valid_out) -> inputs_bus2/4  "VINP_ABSFaultValid_flg_InHAL"
  Bus Selector2/1 -> BrkLampOn/1(signal_in)  "<VHAL_BrkLampOn_flg>"
  [BHAL_outputs] -> Bus Selector2/1
  Logical Operator1/1 -> BrkLampOn/2(signal_valid_in)
  BrkLampOn/1(signal_out) -> inputs_bus2/5  "VINP_BrkLampOn_flg_InHAL"
  BrkLampOn/2(signal_valid_out) -> inputs_bus2/6  "VINP_BrkLampOnValid_flg_InHAL"
  Bus Selector2/2 -> Logical Operator1/1  "<VHAL_BrkLampSigInvalid_flg>"
  Bus Selector14/1 -> EBDFault/1(signal_in)  "<VHAL_EBDFault_flg>"
  [BHAL_outputs] -> Bus Selector14/1
  EBDFault/1(signal_out) -> inputs_bus2/8  "VINP_EBDFault_flg_InHAL"
  EBDFault/2(signal_valid_out) -> inputs_bus2/9  "VINP_EBDFaultValid_flg_InHAL"
  Bus Selector15/1 -> ESCActive/1(signal_in)  "<VHAL_ESCActive_flg>"
  [BHAL_outputs] -> Bus Selector15/1
  ESCActive/1(signal_out) -> inputs_bus2/10  "VINP_ESCActive_flg_InHAL"
  ESCActive/2(signal_valid_out) -> inputs_bus2/11  "VINP_ESCActiveValid_flg_InHAL"
  Bus Selector4/1 -> ESCFault/1(signal_in)  "<VHAL_ESCFault_flg>"
  [BHAL_outputs] -> Bus Selector4/1
  ESCFault/1(signal_out) -> inputs_bus2/12  "VINP_ESCFault_flg_InHAL"
  ESCFault/2(signal_valid_out) -> inputs_bus2/13  "VINP_ESCFaultValid_flg_InHAL"
  Bus Selector17/1 -> TCSActive/1(signal_in)  "<VHAL_TCSActive_flg>"
  [BHAL_outputs] -> Bus Selector17/1
  TCSActive/1(signal_out) -> inputs_bus2/14  "VINP_TCSActive_flg_InHAL"
  TCSActive/2(signal_valid_out) -> inputs_bus2/15  "VINP_TCSActiveValid_flg_InHAL"
  Bus Selector6/1 -> TCSEnabled/1(signal_in)  "<VHAL_TCSEnabled_flg>"
  [BHAL_outputs] -> Bus Selector6/1
  TCSEnabled/1(signal_out) -> inputs_bus2/16  "VINP_TCSEnabled_flg_InHAL"
  TCSEnabled/2(signal_valid_out) -> inputs_bus2/17  "VINP_TCSEnabledValid_flg_InHAL"
  Bus Selector19/1 -> TCSFault/1(signal_in)  "<VHAL_TCSFault_flg>"
  [BHAL_outputs] -> Bus Selector19/1
  TCSFault/1(signal_out) -> inputs_bus2/18  "VINP_TCSFault_flg_InHAL"
  TCSFault/2(signal_valid_out) -> inputs_bus2/19  "VINP_TCSFaultValid_flg_InHAL"
  VehicleSpeed/1(signal_out) -> inputs_bus2/24  "VINP_VehSpd_kph_InHAL"
  VehicleSpeed/2(signal_valid_out) -> inputs_bus2/25  "VINP_VehSpdValid_flg_InHAL"
  Bus Selector8/2 -> Logical Operator3/1  "<VHAL_VehSpdInvalid_flg>"
  [BHAL_outputs] -> Bus Selector8/1
  Bus Selector8/1 -> VehicleSpeed/1(signal_in)  "<VHAL_VehSpd_kph>"
  Logical Operator3/1 -> VehicleSpeed/2(signal_valid_in)
  Bus Selector23/1 -> YawRate/3(msg_valid)  "<VINP_CANMsgValidABS4_flg>"
  [CANvalid_bus] -> Bus Selector23/1
  YawRate/1(signal_out) -> inputs_bus2/27  "VINP_YawRate_degps_InHAL"
  YawRate/2(signal_valid_out) -> inputs_bus2/28  "VINP_YawRateValid_flg_InHAL"
  Bus Selector24/2 -> Logical Operator4/1  "<VHAL_YawRateInvalid_flg>"
  [BHAL_outputs] -> Bus Selector24/1
  Logical Operator4/1 -> YawRate/2(signal_valid_in)
  Bus Selector24/1 -> Gain/1  "<VHAL_YawRate_degps>"
  CANvalid_bus/1 -> [CANvalid_bus]
  Logical Operator9/1 -> ABSActive/2(msg_valid)
  Bus Selector11/2 -> Logical Operator9/2  "<VINP_CANMsgUpdatedABS2_flg>"
  Data Store Read3/1 -> Logical Operator5/1
  Data Store Read1/1 -> Logical Operator5/2
  Data Store Read6/1 -> Logical Operator8/2
  Data Store Read5/1 -> Logical Operator8/3
  Logical Operator8/1 -> BrkLampOn/4(Active_fault_flg)
  Data Store Read7/1 -> Logical Operator8/1
  BrkLampOn/3(raw_signal_valid_out) -> inputs_bus2/7  "VINP_BrkLampOnSigOk_flg_InHAL"
  Data Store Read21/1 -> Logical Operator24/2
  Data Store Read20/1 -> Logical Operator24/3
  Data Store Read22/1 -> Logical Operator24/1
  VehicleSpeed/3(raw_signal_valid_out) -> inputs_bus2/26  "VINP_VehSpdSigOk_flg_InHAL"
  Data Store Read26/1 -> Logical Operator26/1
  Data Store Read25/1 -> Logical Operator26/2
  Logical Operator26/1 -> YawRate/4(Active_fault_flg)
  YawRate/3(raw_signal_valid_out) -> inputs_bus2/29  "VINP_YawRateSigOk_flg_InHAL"
  Bus Selector13/1 -> LatAccel/3(msg_valid)  "<VINP_CANMsgValidABS4_flg>"
  [CANvalid_bus] -> Bus Selector13/1
  Bus Selector22/2 -> Logical Operator25/1  "<VHAL_LatAccelInvalid_flg>"
  [BHAL_outputs] -> Bus Selector22/1
  Logical Operator25/1 -> LatAccel/2(signal_valid_in)
  Bus Selector22/1 -> Gain1/1  "<VHAL_LatAccel_G>"
  Data Store Read28/1 -> Logical Operator35/1
  Data Store Read23/1 -> Logical Operator35/2
  Bus Selector25/1 -> LongAccel/3(msg_valid)  "<VINP_CANMsgValidABS4_flg>"
  [CANvalid_bus] -> Bus Selector25/1
  Bus Selector26/2 -> Logical Operator28/1  "<VHAL_LongAccelInvalid_flg>"
  [BHAL_outputs] -> Bus Selector26/1
  Logical Operator28/1 -> LongAccel/2(signal_valid_in)
  Bus Selector26/1 -> LongAccel/1(signal_in)  "<VHAL_LongAccel_G>"
  Data Store Read29/1 -> Logical Operator2/1
  Data Store Read27/1 -> Logical Operator2/2
  Bus Selector27/1 -> MCPressure/3(msg_valid)  "<VINP_CANMsgValidABS4_flg>"
  [CANvalid_bus] -> Bus Selector27/1
  Bus Selector28/2 -> Logical Operator30/1  "<VHAL_MCPressureInvalid_flg>"
  [BHAL_outputs] -> Bus Selector28/1
  Logical Operator30/1 -> MCPressure/2(signal_valid_in)
  Bus Selector28/1 -> MCPressure/1(signal_in)  "<VHAL_MCPressure_bar>"
  Data Store Read30/1 -> Logical Operator27/1
  Data Store Read24/1 -> Logical Operator27/2
  LatAccel/1(signal_out) -> inputs_bus2/30  "VINP_LatAccel_G_InHAL"
  LatAccel/2(signal_valid_out) -> inputs_bus2/31  "VINP_LatAccelValid_flg_InHAL"
  LatAccel/3(raw_signal_valid_out) -> inputs_bus2/32  "VINP_LatAccelSigOk_flg_InHAL"
  LongAccel/1(signal_out) -> inputs_bus2/33  "VINP_LongAccel_G_InHAL"
  LongAccel/2(signal_valid_out) -> inputs_bus2/34  "VINP_LongAccelValid_flg_InHAL"
  LongAccel/3(raw_signal_valid_out) -> inputs_bus2/35  "VINP_LongAccelSigOk_flg_InHAL"
  MCPressure/1(signal_out) -> inputs_bus2/36  "VINP_MCPressure_bar_InHAL"
  MCPressure/2(signal_valid_out) -> inputs_bus2/37  "VINP_MCPressureValid_flg_InHAL"
  MCPressure/3(raw_signal_valid_out) -> inputs_bus2/38  "VINP_MCPressureSigOk_flg_InHAL"
  Bus Selector11/3 -> Logical Operator9/3  "<VINP_CANMsgCheckedABS2_flg>"
  Data Store Read31/1 -> Logical Operator5/3
  Bus Selector9/1 -> Logical Operator7/1  "<VINP_CANMsgValidABS2_flg>"
  [CANvalid_bus] -> Bus Selector9/1
  Logical Operator6/1 -> ABSFault/3(Active_fault_flg)
  Logical Operator7/1 -> ABSFault/2(msg_valid)
  Bus Selector9/2 -> Logical Operator7/2  "<VINP_CANMsgUpdatedABS2_flg>"
  Data Store Read4/1 -> Logical Operator6/1
  Data Store Read2/1 -> Logical Operator6/2
  Bus Selector9/3 -> Logical Operator7/3  "<VINP_CANMsgCheckedABS2_flg>"
  Data Store Read32/1 -> Logical Operator6/3
  Bus Selector12/1 -> Logical Operator10/1  "<VINP_CANMsgValidABS2_flg>"
  [CANvalid_bus] -> Bus Selector12/1
  Logical Operator10/1 -> BrkLampOn/3(msg_valid)
  Bus Selector12/2 -> Logical Operator10/2  "<VINP_CANMsgUpdatedABS2_flg>"
  Bus Selector12/3 -> Logical Operator10/3  "<VINP_CANMsgCheckedABS2_flg>"
  Data Store Read33/1 -> Logical Operator8/4
  Bus Selector3/1 -> Logical Operator12/1  "<VINP_CANMsgValidABS2_flg>"
  [CANvalid_bus] -> Bus Selector3/1
  Logical Operator11/1 -> EBDFault/3(Active_fault_flg)
  Logical Operator12/1 -> EBDFault/2(msg_valid)
  Bus Selector3/2 -> Logical Operator12/2  "<VINP_CANMsgUpdatedABS2_flg>"
  Data Store Read34/1 -> Logical Operator11/1
  Data Store Read8/1 -> Logical Operator11/2
  Bus Selector3/3 -> Logical Operator12/3  "<VINP_CANMsgCheckedABS2_flg>"
  Data Store Read9/1 -> Logical Operator11/3
  Bus Selector21/1 -> Logical Operator14/1  "<VINP_CANMsgValidABS2_flg>"
  [CANvalid_bus] -> Bus Selector21/1
  Logical Operator13/1 -> ESCActive/3(Active_fault_flg)
  Logical Operator14/1 -> ESCActive/2(msg_valid)
  Bus Selector21/2 -> Logical Operator14/2  "<VINP_CANMsgUpdatedABS2_flg>"
  Data Store Read10/1 -> Logical Operator13/1
  Data Store Read11/1 -> Logical Operator13/2
  Bus Selector21/3 -> Logical Operator14/3  "<VINP_CANMsgCheckedABS2_flg>"
  Data Store Read35/1 -> Logical Operator13/3
  Bus Selector16/1 -> Logical Operator16/1  "<VINP_CANMsgValidABS2_flg>"
  [CANvalid_bus] -> Bus Selector16/1
  Logical Operator15/1 -> ESCFault/3(Active_fault_flg)
  Logical Operator16/1 -> ESCFault/2(msg_valid)
  Bus Selector16/2 -> Logical Operator16/2  "<VINP_CANMsgUpdatedABS2_flg>"
  Data Store Read12/1 -> Logical Operator15/1
  Data Store Read13/1 -> Logical Operator15/2
  Bus Selector16/3 -> Logical Operator16/3  "<VINP_CANMsgCheckedABS2_flg>"
  Data Store Read36/1 -> Logical Operator15/3
  Bus Selector5/1 -> Logical Operator18/1  "<VINP_CANMsgValidABS2_flg>"
  [CANvalid_bus] -> Bus Selector5/1
  Logical Operator17/1 -> TCSActive/3(Active_fault_flg)
  Logical Operator18/1 -> TCSActive/2(msg_valid)
  Bus Selector5/2 -> Logical Operator18/2  "<VINP_CANMsgUpdatedABS2_flg>"
  Data Store Read14/1 -> Logical Operator17/1
  Data Store Read15/1 -> Logical Operator17/2
  Bus Selector5/3 -> Logical Operator18/3  "<VINP_CANMsgCheckedABS2_flg>"
  Data Store Read37/1 -> Logical Operator17/3
  Bus Selector18/1 -> Logical Operator20/1  "<VINP_CANMsgValidABS2_flg>"
  [CANvalid_bus] -> Bus Selector18/1
  Logical Operator19/1 -> TCSEnabled/3(Active_fault_flg)
  Logical Operator20/1 -> TCSEnabled/2(msg_valid)
  Bus Selector18/2 -> Logical Operator20/2  "<VINP_CANMsgUpdatedABS2_flg>"
  Data Store Read16/1 -> Logical Operator19/1
  Data Store Read17/1 -> Logical Operator19/2
  Bus Selector18/3 -> Logical Operator20/3  "<VINP_CANMsgCheckedABS2_flg>"
  Data Store Read38/1 -> Logical Operator19/3
  Bus Selector7/1 -> Logical Operator22/1  "<VINP_CANMsgValidABS2_flg>"
  [CANvalid_bus] -> Bus Selector7/1
  Logical Operator21/1 -> TCSFault/3(Active_fault_flg)
  Logical Operator22/1 -> TCSFault/2(msg_valid)
  Bus Selector7/2 -> Logical Operator22/2  "<VINP_CANMsgUpdatedABS2_flg>"
  Data Store Read18/1 -> Logical Operator21/1
  Data Store Read19/1 -> Logical Operator21/2
  Bus Selector7/3 -> Logical Operator22/3  "<VINP_CANMsgCheckedABS2_flg>"
  Data Store Read39/1 -> Logical Operator21/3
  Bus Selector20/1 -> Logical Operator23/1  "<VINP_CANMsgValidABS2_flg>"
  [CANvalid_bus] -> Bus Selector20/1
  Logical Operator23/1 -> VehicleSpeed/3(msg_valid)
  Bus Selector20/2 -> Logical Operator23/2  "<VINP_CANMsgUpdatedABS2_flg>"
  Bus Selector20/3 -> Logical Operator23/3  "<VINP_CANMsgCheckedABS2_flg>"
  Logical Operator24/1 -> VehicleSpeed/4(Active_fault_flg)
  Data Store Read40/1 -> Logical Operator24/4
  Bus Selector29/1 -> HSAActive/1(signal_in)  "<VHAL_HSAActive_flg>"
  [BHAL_outputs] -> Bus Selector29/1
  HSAActive/1(signal_out) -> inputs_bus2/20  "VINP_HSAActive_flg_InHAL"
  HSAActive/2(signal_valid_out) -> inputs_bus2/21  "VINP_HSAActiveValid_flg_InHAL"
  Bus Selector30/1 -> Logical Operator32/1  "<VINP_CANMsgValidABS2_flg>"
  [CANvalid_bus] -> Bus Selector30/1
  Logical Operator31/1 -> HSAActive/3(Active_fault_flg)
  Logical Operator32/1 -> HSAActive/2(msg_valid)
  Bus Selector30/2 -> Logical Operator32/2  "<VINP_CANMsgUpdatedABS2_flg>"
  Data Store Read41/1 -> Logical Operator31/1
  Data Store Read42/1 -> Logical Operator31/2
  Bus Selector30/3 -> Logical Operator32/3  "<VINP_CANMsgCheckedABS2_flg>"
  Data Store Read43/1 -> Logical Operator31/3
  Bus Selector31/1 -> HSAFault/1(signal_in)  "<VHAL_HSAFault_flg>"
  [BHAL_outputs] -> Bus Selector31/1
  HSAFault/1(signal_out) -> inputs_bus2/22  "VINP_HSAFault_flg_InHAL"
  HSAFault/2(signal_valid_out) -> inputs_bus2/23  "VINP_HSAFaultValid_flg_InHAL"
  Bus Selector32/1 -> Logical Operator34/1  "<VINP_CANMsgValidABS2_flg>"
  [CANvalid_bus] -> Bus Selector32/1
  Logical Operator33/1 -> HSAFault/3(Active_fault_flg)
  Logical Operator34/1 -> HSAFault/2(msg_valid)
  Bus Selector32/2 -> Logical Operator34/2  "<VINP_CANMsgUpdatedABS2_flg>"
  Data Store Read44/1 -> Logical Operator33/1
  Data Store Read45/1 -> Logical Operator33/2
  Bus Selector32/3 -> Logical Operator34/3  "<VINP_CANMsgCheckedABS2_flg>"
  Data Store Read46/1 -> Logical Operator33/3
  Logical Operator35/1 -> LatAccel/4(Active_fault_flg)
  Logical Operator2/1 -> LongAccel/4(Active_fault_flg)
  Logical Operator27/1 -> MCPressure/4(Active_fault_flg)
  Bus Selector33/1 -> Logical Operator37/1  "<VINP_CANMsgValidABS1_flg>"
  [CANvalid_bus] -> Bus Selector33/1
  Bus Selector34/2 -> Logical Operator36/1  "<VHAL_SurfaceGradeInvalid_flg>"
  [BHAL_outputs] -> Bus Selector34/1
  Logical Operator36/1 -> SurfaceGrade/2(signal_valid_in)
  Bus Selector34/1 -> SurfaceGrade/1(signal_in)  "<VHAL_SurfaceGrade_deg>"
  Data Store Read48/1 -> Logical Operator38/1
  Data Store Read47/1 -> Logical Operator38/2
  SurfaceGrade/1(signal_out) -> inputs_bus2/39  "VINP_SurfaceGrade_deg_InHAL"
  SurfaceGrade/2(signal_valid_out) -> inputs_bus2/40  "VINP_SurfaceGradeValid_flg_InHAL"
  SurfaceGrade/3(raw_signal_valid_out) -> inputs_bus2/41  "VINP_SurfaceGradeSigOk_flg_InHAL"
  Logical Operator38/1 -> SurfaceGrade/4(Active_fault_flg)
  Logical Operator37/1 -> SurfaceGrade/3(msg_valid)
  Bus Selector33/2 -> Logical Operator37/2  "<VINP_CANMsgUpdatedABS1_flg>"
  Data Store Read49/1 -> Logical Operator38/3
  Bus Selector35/1 -> Logical Operator39/1  "<VINP_CANMsgValidABS1_flg>"
  [CANvalid_bus] -> Bus Selector35/1
  Bus Selector36/2 -> Logical Operator29/1  "<VHAL_BrakBoosterPresInvalid_flg>"
  [BHAL_outputs] -> Bus Selector36/1
  Logical Operator29/1 -> BrakBoosterPres/2(signal_valid_in)
  Bus Selector36/1 -> BrakBoosterPres/1(signal_in)  "<VHAL_BrakBoosterPres_kPa>"
  Data Store Read51/1 -> Logical Operator40/1
  Data Store Read50/1 -> Logical Operator40/2
  BrakBoosterPres/1(signal_out) -> inputs_bus2/42  "VINP_BrakBoosterPres_kPa_InHAL"
  BrakBoosterPres/2(signal_valid_out) -> inputs_bus2/43, [VINP_BrakBoosterPresValid_flg_InHAL]  "VINP_BrakBoosterPresValid_flg_InHAL"
  BrakBoosterPres/3(raw_signal_valid_out) -> inputs_bus2/44, [VINP_BrakBoosterPresSigOk_flg_InHAL]  "VINP_BrakBoosterPresSigOk_flg_InHAL"
  Logical Operator40/1 -> BrakBoosterPres/4(Active_fault_flg)
  Logical Operator39/1 -> BrakBoosterPres/3(msg_valid)
  Bus Selector35/2 -> Logical Operator39/2  "<VINP_CANMsgUpdatedABS1_flg>"
  Data Store Read52/1 -> Logical Operator40/3
  Bus Selector37/1 -> LowBrakBoosterPres/1(signal_in)  "<VHAL_LowBrakBoosterPres_flg>"
  [BHAL_outputs] -> Bus Selector37/1
  LowBrakBoosterPres/1(signal_out) -> inputs_bus2/45  "VINP_LowBrakBoosterPres_flg_InHAL"
  LowBrakBoosterPres/2(signal_valid_out) -> inputs_bus2/46  "VINP_LowBrakBoosterPresValid_flg_InHAL"
  Logical Operator41/1 -> LowBrakBoosterPres/3(Active_fault_flg)
  Data Store Read53/1 -> Logical Operator41/1
  [CANvalid_bus] -> Bus Selector38/1
  Logical Operator42/1 -> LowBrakBoosterPres/2(msg_valid)
  Bus Selector38/1 -> Logical Operator42/1  "<VINP_CANMsgValidABS1_flg>"
  Bus Selector38/2 -> Logical Operator42/2  "<VINP_CANMsgUpdatedABS1_flg>"
  Data Store Read54/1 -> Logical Operator41/2
  Data Store Read55/1 -> Logical Operator41/3
  [VINP_BrakBoosterPresValid_flg_InHAL] -> Logical Operator42/3
  [VINP_BrakBoosterPresSigOk_flg_InHAL] -> Logical Operator42/4
  Bus Selector40/1 -> LFWheelSpeed/3(msg_valid)  "<VINP_CANMsgValidABS3_flg>"
  [CANvalid_bus] -> Bus Selector40/1
  Bus Selector42/2 -> Logical Operator44/1  "<VHAL_LFWheelSpeedInvalid_flg>"
  [BHAL_outputs] -> Bus Selector42/1
  Logical Operator44/1 -> LFWheelSpeed/2(signal_valid_in)
  Bus Selector42/1 -> LFWheelSpeed/1(signal_in)  "<VHAL_LFWheelSpeed_kph>"
  Data Store Read59/1 -> Logical Operator46/1
  Data Store Read58/1 -> Logical Operator46/2
  LFWheelSpeed/1(signal_out) -> inputs_bus2/47  "VINP_LFWheelSpeed_kph_InHAL"
  LFWheelSpeed/2(signal_valid_out) -> inputs_bus2/48  "VINP_LFWheelSpeedValid_flg_InHAL"
  LFWheelSpeed/3(raw_signal_valid_out) -> inputs_bus2/49  "VINP_LFWheelSpeedSigOk_flg_InHAL"
  Logical Operator46/1 -> LFWheelSpeed/4(Active_fault_flg)
  Bus Selector43/1 -> LRWheelSpeed/3(msg_valid)  "<VINP_CANMsgValidABS3_flg>"
  [CANvalid_bus] -> Bus Selector43/1
  Bus Selector44/2 -> Logical Operator47/1  "<VHAL_LRWheelSpeedInvalid_flg>"
  [BHAL_outputs] -> Bus Selector44/1
  Logical Operator47/1 -> LRWheelSpeed/2(signal_valid_in)
  Bus Selector44/1 -> LRWheelSpeed/1(signal_in)  "<VHAL_LRWheelSpeed_kph>"
  Data Store Read61/1 -> Logical Operator48/1
  Data Store Read60/1 -> Logical Operator48/2
  LRWheelSpeed/1(signal_out) -> inputs_bus2/50  "VINP_LRWheelSpeed_kph_InHAL"
  LRWheelSpeed/2(signal_valid_out) -> inputs_bus2/51  "VINP_LRWheelSpeedValid_flg_InHAL"
  LRWheelSpeed/3(raw_signal_valid_out) -> inputs_bus2/52  "VINP_LRWheelSpeedSigOk_flg_InHAL"
  Logical Operator48/1 -> LRWheelSpeed/4(Active_fault_flg)
  Bus Selector45/1 -> RFWheelSpeed/3(msg_valid)  "<VINP_CANMsgValidABS3_flg>"
  [CANvalid_bus] -> Bus Selector45/1
  Bus Selector46/2 -> Logical Operator49/1  "<VHAL_RFWheelSpeedInvalid_flg>"
  [BHAL_outputs] -> Bus Selector46/1
  Logical Operator49/1 -> RFWheelSpeed/2(signal_valid_in)
  Bus Selector46/1 -> RFWheelSpeed/1(signal_in)  "<VHAL_RFWheelSpeed_kph>"
  Data Store Read63/1 -> Logical Operator50/1
  Data Store Read62/1 -> Logical Operator50/2
  RFWheelSpeed/1(signal_out) -> inputs_bus2/53  "VINP_RFWheelSpeed_kph_InHAL"
  RFWheelSpeed/2(signal_valid_out) -> inputs_bus2/54  "VINP_RFWheelSpeedValid_flg_InHAL"
  RFWheelSpeed/3(raw_signal_valid_out) -> inputs_bus2/55  "VINP_RFWheelSpeedSigOk_flg_InHAL"
  Logical Operator50/1 -> RFWheelSpeed/4(Active_fault_flg)
  Bus Selector47/1 -> RRWheelSpeed/3(msg_valid)  "<VINP_CANMsgValidABS3_flg>"
  [CANvalid_bus] -> Bus Selector47/1
  Bus Selector48/2 -> Logical Operator51/1  "<VHAL_RRWheelSpeedInvalid_flg>"
  [BHAL_outputs] -> Bus Selector48/1
  Logical Operator51/1 -> RRWheelSpeed/2(signal_valid_in)
  Bus Selector48/1 -> RRWheelSpeed/1(signal_in)  "<VHAL_RRWheelSpeed_kph>"
  Data Store Read65/1 -> Logical Operator52/1
  Data Store Read64/1 -> Logical Operator52/2
  RRWheelSpeed/1(signal_out) -> inputs_bus2/56  "VINP_RRWheelSpeed_kph_InHAL"
  RRWheelSpeed/2(signal_valid_out) -> inputs_bus2/57  "VINP_RRWheelSpeedValid_flg_InHAL"
  RRWheelSpeed/3(raw_signal_valid_out) -> inputs_bus2/58  "VINP_RRWheelSpeedSigOk_flg_InHAL"
  Logical Operator52/1 -> RRWheelSpeed/4(Active_fault_flg)
  Gain/1 -> YawRate/1(signal_in)
  Gain1/1 -> LatAccel/1(signal_in)

== system inp/INP/AC_signals ==
  BHAL_outputs : Inport  OutDataTypeStr=Bus: BHAL_outputs
  CANvalid_bus : Inport  Port=2
  Bus Selector8 : BusSelector  OutputSignals=VHAL_OutsideTemp_degC,VHAL_OutsideTempInvalid_flg
  Bus Selector9 : BusSelector  OutputSignals=VINP_CANMsgValidAC1_flg
  Data Store Read13 : DataStoreRead  DataStoreName=VDDML_AC1CANCommuFault_AF_flg
  Data Store Read14 : DataStoreRead  DataStoreName=VDDML_CANOutsideTempSigFault_AF_flg
  Logical Operator14 : Logic  Tag=struct('inputs','1','comment',struct('text','','placement',1),'logdata',struct('maxnumvals',inf,'plotchannels',[],'stepsize',0,'downsampling',1,'loggingmode',3,'channels',1),'operator','NOT','output',struct('class','default','type','Bool8','description','','address','','unit','','name','$S_$B'),'date',731430.46749816)  Operator=NOT
  Logical Operator6 : Logic  Tag=struct('inputs','1','comment',struct('text','','placement',1),'logdata',struct('maxnumvals',inf,'plotchannels',[],'stepsize',0,'downsampling',1,'loggingmode',3,'channels',1),'operator','NOT','output',struct('class','default','type','Bool8','description','','address','','unit','','name','$S_$B'),'date',731430.46749816)  Operator=OR
  OutsideTemp : Subsystem
      settings: RTWSystemCode=Inline
  inputs_bus2 : BusCreator  Inputs=3
  AC_signal_bus : Outport
  wires:
  inputs_bus2/1 -> AC_signal_bus/1
  BHAL_outputs/1 -> [BHAL_outputs]
  CANvalid_bus/1 -> [CANvalid_bus]
  [BHAL_outputs] -> Bus Selector8/1
  Bus Selector8/1 -> OutsideTemp/1(signal_in)  "<VHAL_OutsideTemp_degC>"
  Bus Selector8/2 -> Logical Operator14/1  "<VHAL_OutsideTempInvalid_flg>"
  Logical Operator14/1 -> OutsideTemp/2(signal_valid_in)
  Data Store Read14/1 -> Logical Operator6/1
  Data Store Read13/1 -> Logical Operator6/2
  OutsideTemp/1(signal_out) -> inputs_bus2/1  "VINP_OutsideTemp_degC_InHAL"
  OutsideTemp/2(signal_valid_out) -> inputs_bus2/2  "VINP_OutsideTempValid_flg_InHAL"
  OutsideTemp/3(raw_signal_valid_out) -> inputs_bus2/3  "VINP_OutsideTempSigOk_flg_InHAL"
  [CANvalid_bus] -> Bus Selector9/1
  Bus Selector9/1 -> OutsideTemp/3(msg_valid)  "<VINP_CANMsgValidAC1_flg>"
  Logical Operator6/1 -> OutsideTemp/4(Active_fault_flg)
